simulink model "he6_cres_correlator"  (R2012b) (continued)
== system he6_cres_correlator == (continued)
      mask init:
      | pipeline_init(gcb,'latency',latency);
      settings: AttributesFormatString=Latency = %<latency>
      mask Display:
      | port_label('input', 1, 'd');
      | port_label('output', 1, 'q');
      | disp(['Z^-', num2str(latency)]);
  pipeline22 : Subsystem  AncestorBlock=casper_library_delays/pipeline  mask(pipeline){latency=1}
      mask help:
      | An explicitly laid-out delay line to avoid mapping to SRL16s
      | for use in pipelining to help achieve timing closure.
      mask init:
      | pipeline_init(gcb,'latency',latency);
      settings: AttributesFormatString=Latency = %<latency>
      mask Display:
      | port_label('input', 1, 'd');
      | port_label('output', 1, 'q');
      | disp(['Z^-', num2str(latency)]);
  pipeline23 : Subsystem  AncestorBlock=casper_library_delays/pipeline  mask(pipeline){latency=1}
      mask help:
      | An explicitly laid-out delay line to avoid mapping to SRL16s
      | for use in pipelining to help achieve timing closure.
      mask init:
      | pipeline_init(gcb,'latency',latency);
      settings: AttributesFormatString=Latency = %<latency>
      mask Display:
      | port_label('input', 1, 'd');
      | port_label('output', 1, 'q');
      | disp(['Z^-', num2str(latency)]);
  pipeline24 : Subsystem  AncestorBlock=casper_library_delays/pipeline  mask(pipeline){latency=1}
      mask help:
      | An explicitly laid-out delay line to avoid mapping to SRL16s
      | for use in pipelining to help achieve timing closure.
      mask init:
      | pipeline_init(gcb,'latency',latency);
      settings: AttributesFormatString=Latency = %<latency>
      mask Display:
      | port_label('input', 1, 'd');
      | port_label('output', 1, 'q');
      | disp(['Z^-', num2str(latency)]);
  pipeline25 : Subsystem  AncestorBlock=casper_library_delays/pipeline  mask(pipeline){latency=1}
      mask help:
      | An explicitly laid-out delay line to avoid mapping to SRL16s
      | for use in pipelining to help achieve timing closure.
      mask init:
      | pipeline_init(gcb,'latency',latency);
      settings: AttributesFormatString=Latency = %<latency>
      mask Display:
      | port_label('input', 1, 'd');
      | port_label('output', 1, 'q');
      | disp(['Z^-', num2str(latency)]);
  pipeline26 : Subsystem  AncestorBlock=casper_library_delays/pipeline  mask(pipeline){latency=1}
      mask help:
      | An explicitly laid-out delay line to avoid mapping to SRL16s
      | for use in pipelining to help achieve timing closure.
      mask init:
      | pipeline_init(gcb,'latency',latency);
      settings: AttributesFormatString=Latency = %<latency>
      mask Display:
      | port_label('input', 1, 'd');
      | port_label('output', 1, 'q');
      | disp(['Z^-', num2str(latency)]);
  pipeline27 : Subsystem  AncestorBlock=casper_library_delays/pipeline  mask(pipeline){latency=1}
      mask help:
      | An explicitly laid-out delay line to avoid mapping to SRL16s
      | for use in pipelining to help achieve timing closure.
      mask init:
      | pipeline_init(gcb,'latency',latency);
      settings: AttributesFormatString=Latency = %<latency>
      mask Display:
      | port_label('input', 1, 'd');
      | port_label('output', 1, 'q');
      | disp(['Z^-', num2str(latency)]);
  pipeline28 : Subsystem  AncestorBlock=casper_library_delays/pipeline  mask(pipeline){latency=1}
      mask help:
      | An explicitly laid-out delay line to avoid mapping to SRL16s
      | for use in pipelining to help achieve timing closure.
      mask init:
      | pipeline_init(gcb,'latency',latency);
      settings: AttributesFormatString=Latency = %<latency>
      mask Display:
      | port_label('input', 1, 'd');
      | port_label('output', 1, 'q');
      | disp(['Z^-', num2str(latency)]);
  pipeline29 : Subsystem  AncestorBlock=casper_library_delays/pipeline  mask(pipeline){latency=1}
      mask help:
      | An explicitly laid-out delay line to avoid mapping to SRL16s
      | for use in pipelining to help achieve timing closure.
      mask init:
      | pipeline_init(gcb,'latency',latency);
      settings: AttributesFormatString=Latency = %<latency>
      mask Display:
      | port_label('input', 1, 'd');
      | port_label('output', 1, 'q');
      | disp(['Z^-', num2str(latency)]);
  pipeline3 : Subsystem  AncestorBlock=casper_library_delays/pipeline  mask(pipeline){latency=1}
      mask help:
      | An explicitly laid-out delay line to avoid mapping to SRL16s
      | for use in pipelining to help achieve timing closure.
      mask init:
      | pipeline_init(gcb,'latency',latency);
      settings: AttributesFormatString=Latency = %<latency>
      mask Display:
      | port_label('input', 1, 'd');
      | port_label('output', 1, 'q');
      | disp(['Z^-', num2str(latency)]);
  pipeline30 : Subsystem  AncestorBlock=casper_library_delays/pipeline  mask(pipeline){latency=1}
      mask help:
      | An explicitly laid-out delay line to avoid mapping to SRL16s
      | for use in pipelining to help achieve timing closure.
      mask init:
      | pipeline_init(gcb,'latency',latency);
      settings: AttributesFormatString=Latency = %<latency>
      mask Display:
      | port_label('input', 1, 'd');
      | port_label('output', 1, 'q');
      | disp(['Z^-', num2str(latency)]);
  pipeline31 : Subsystem  AncestorBlock=casper_library_delays/pipeline  mask(pipeline){latency=1}
      mask help:
      | An explicitly laid-out delay line to avoid mapping to SRL16s
      | for use in pipelining to help achieve timing closure.
      mask init:
      | pipeline_init(gcb,'latency',latency);
      settings: AttributesFormatString=Latency = %<latency>
      mask Display:
      | port_label('input', 1, 'd');
      | port_label('output', 1, 'q');
      | disp(['Z^-', num2str(latency)]);
  pipeline32 : Subsystem  AncestorBlock=casper_library_delays/pipeline  mask(pipeline){latency=1}
      mask help:
      | An explicitly laid-out delay line to avoid mapping to SRL16s
      | for use in pipelining to help achieve timing closure.
      mask init:
      | pipeline_init(gcb,'latency',latency);
      settings: AttributesFormatString=Latency = %<latency>
      mask Display:
      | port_label('input', 1, 'd');
      | port_label('output', 1, 'q');
      | disp(['Z^-', num2str(latency)]);
  pipeline33 : Subsystem  AncestorBlock=casper_library_delays/pipeline  mask(pipeline){latency=1}
      mask help:
      | An explicitly laid-out delay line to avoid mapping to SRL16s
      | for use in pipelining to help achieve timing closure.
      mask init:
      | pipeline_init(gcb,'latency',latency);
      settings: AttributesFormatString=Latency = %<latency>
      mask Display:
      | port_label('input', 1, 'd');
      | port_label('output', 1, 'q');
      | disp(['Z^-', num2str(latency)]);
  pipeline34 : Subsystem  AncestorBlock=casper_library_delays/pipeline  mask(pipeline){latency=1}
      mask help:
      | An explicitly laid-out delay line to avoid mapping to SRL16s
      | for use in pipelining to help achieve timing closure.
      mask init:
      | pipeline_init(gcb,'latency',latency);
      settings: AttributesFormatString=Latency = %<latency>
      mask Display:
      | port_label('input', 1, 'd');
      | port_label('output', 1, 'q');
      | disp(['Z^-', num2str(latency)]);
  pipeline35 : Subsystem  AncestorBlock=casper_library_delays/pipeline  mask(pipeline){latency=1}
      mask help:
      | An explicitly laid-out delay line to avoid mapping to SRL16s
      | for use in pipelining to help achieve timing closure.
      mask init:
      | pipeline_init(gcb,'latency',latency);
      settings: AttributesFormatString=Latency = %<latency>
      mask Display:
      | port_label('input', 1, 'd');
      | port_label('output', 1, 'q');
      | disp(['Z^-', num2str(latency)]);
  pipeline36 : Subsystem  AncestorBlock=casper_library_delays/pipeline  mask(pipeline){latency=1}
      mask help:
      | An explicitly laid-out delay line to avoid mapping to SRL16s
      | for use in pipelining to help achieve timing closure.
      mask init:
      | pipeline_init(gcb,'latency',latency);
      settings: AttributesFormatString=Latency = %<latency>
      mask Display:
      | port_label('input', 1, 'd');
      | port_label('output', 1, 'q');
      | disp(['Z^-', num2str(latency)]);
  pipeline37 : Subsystem  AncestorBlock=casper_library_delays/pipeline  mask(pipeline){latency=3}
      mask help:
      | An explicitly laid-out delay line to avoid mapping to SRL16s
      | for use in pipelining to help achieve timing closure.
      mask init:
      | pipeline_init(gcb,'latency',latency);
      settings: AttributesFormatString=Latency = %<latency>
      mask Display:
      | port_label('input', 1, 'd');
      | port_label('output', 1, 'q');
      | disp(['Z^-', num2str(latency)]);
  pipeline4 : Subsystem  AncestorBlock=casper_library_delays/pipeline  mask(pipeline){latency=2}
      mask help:
      | An explicitly laid-out delay line to avoid mapping to SRL16s
      | for use in pipelining to help achieve timing closure.
      mask init:
      | pipeline_init(gcb,'latency',latency);
      settings: AttributesFormatString=Latency = %<latency>
      mask Display:
      | port_label('input', 1, 'd');
      | port_label('output', 1, 'q');
      | disp(['Z^-', num2str(latency)]);
  pipeline6 : Subsystem  AncestorBlock=casper_library_delays/pipeline  mask(pipeline){latency=5}
      mask help:
      | An explicitly laid-out delay line to avoid mapping to SRL16s
      | for use in pipelining to help achieve timing closure.
      mask init:
      | pipeline_init(gcb,'latency',latency);
      settings: AttributesFormatString=Latency = %<latency>
      mask Display:
      | port_label('input', 1, 'd');
      | port_label('output', 1, 'q');
      | disp(['Z^-', num2str(latency)]);
  pipeline7 : Subsystem  AncestorBlock=casper_library_delays/pipeline  mask(pipeline){latency=1}
      mask help:
      | An explicitly laid-out delay line to avoid mapping to SRL16s
      | for use in pipelining to help achieve timing closure.
      mask init:
      | pipeline_init(gcb,'latency',latency);
      settings: AttributesFormatString=Latency = %<latency>
      mask Display:
      | port_label('input', 1, 'd');
      | port_label('output', 1, 'q');
      | disp(['Z^-', num2str(latency)]);
  pipeline8 : Subsystem  AncestorBlock=casper_library_delays/pipeline  mask(pipeline){latency=1}
      mask help:
      | An explicitly laid-out delay line to avoid mapping to SRL16s
      | for use in pipelining to help achieve timing closure.
      mask init:
      | pipeline_init(gcb,'latency',latency);
      settings: AttributesFormatString=Latency = %<latency>
      mask Display:
      | port_label('input', 1, 'd');
      | port_label('output', 1, 'q');
      | disp(['Z^-', num2str(latency)]);
  pipeline9 : Subsystem  AncestorBlock=casper_library_delays/pipeline  mask(pipeline){latency=1}
      mask help:
      | An explicitly laid-out delay line to avoid mapping to SRL16s
      | for use in pipelining to help achieve timing closure.
      mask init:
      | pipeline_init(gcb,'latency',latency);
      settings: AttributesFormatString=Latency = %<latency>
      mask Display:
      | port_label('input', 1, 'd');
      | port_label('output', 1, 'q');
      | disp(['Z^-', num2str(latency)]);
  power0 : casper_library_misc/power  BitWidth=18  add_latency=2  mult_latency=3
      link override power_adder: mode=Addition  use_carryin=off  use_carryout=off  en=off  latency=add_latency  precision=User Defined  arith_type=Unsigned  n_bits=BitWidth * 2  bin_pt=BitWidth * 2 - 1  quantization=Truncate  overflow=Wrap  dbl_ovrd=off  use_behavioral_HDL=on  hw_selection=Fabric  pipelined=off  use_rpm=on
      link override power_adder: xl_area=[0, 0, 0, 0, 0, 0, 0]
  power{1..7} (7 alike) : casper_library_misc/power  BitWidth=18  add_latency=2  mult_latency=3
  rsv0_a : xbsIndex_r4/Constant  const=0  gui_display_data_type=Fixed-point  arith_type=Unsigned  n_bits=64  bin_pt=0  preci_type=Single  exp_width=8  frac_width=24  explicit_period=on  period=1  equ=P=C  opselect=C  inp2=PCIN>>17  opr=+  inp1=P  carry=CIN  dbl_ovrd=off  block_type=constant
  rsv1_a : xbsIndex_r4/Constant  const=0  gui_display_data_type=Fixed-point  arith_type=Unsigned  n_bits=64  bin_pt=0  preci_type=Single  exp_width=8  frac_width=24  explicit_period=on  period=1  equ=P=C  opselect=C  inp2=PCIN>>17  opr=+  inp1=P  carry=CIN  dbl_ovrd=off  block_type=constant
  simple_bram_vacc : Subsystem  AncestorBlock=casper_library_accumulators/simple_bram_vacc  mask(simple_bram_vacc){vec_len=512, arith_type=Unsigned, n_bits=16, bin_pt=0}
      mask prompts: vec_len "Vector length:"; arith_type "Output type:"; n_bits "Bit width (output):"; bin_pt "Binary point (output):"
      mask help: This simple vector accumulator is based on a shift register. It outputs its previous accumulated vector after a new_acc pulse is received.
      mask init:
      | simple_bram_vacc_init(gcb, ...
      |     'vec_len', vec_len, ...
      |     'arith_type', arith_type, ...
      |     'n_bits', n_bits, ...
      |     'bin_pt', bin_pt);
      mask TypeOptions arith_type: Signed | Unsigned
  simple_bram_vacc1 : Subsystem  AncestorBlock=casper_library_accumulators/simple_bram_vacc  mask(simple_bram_vacc){vec_len=512, arith_type=Unsigned, n_bits=16, bin_pt=0}
      mask prompts: vec_len "Vector length:"; arith_type "Output type:"; n_bits "Bit width (output):"; bin_pt "Binary point (output):"
      mask help: This simple vector accumulator is based on a shift register. It outputs its previous accumulated vector after a new_acc pulse is received.
      mask init:
      | simple_bram_vacc_init(gcb, ...
      |     'vec_len', vec_len, ...
      |     'arith_type', arith_type, ...
      |     'n_bits', n_bits, ...
      |     'bin_pt', bin_pt);
      mask TypeOptions arith_type: Signed | Unsigned
  simple_bram_vacc2 : Subsystem  AncestorBlock=casper_library_accumulators/simple_bram_vacc  mask(simple_bram_vacc){vec_len=512, arith_type=Unsigned, n_bits=16, bin_pt=0}
      mask prompts: vec_len "Vector length:"; arith_type "Output type:"; n_bits "Bit width (output):"; bin_pt "Binary point (output):"
      mask help: This simple vector accumulator is based on a shift register. It outputs its previous accumulated vector after a new_acc pulse is received.
      mask init:
      | simple_bram_vacc_init(gcb, ...
      |     'vec_len', vec_len, ...
      |     'arith_type', arith_type, ...
      |     'n_bits', n_bits, ...
      |     'bin_pt', bin_pt);
      mask TypeOptions arith_type: Signed | Unsigned
  simple_bram_vacc3 : Subsystem  AncestorBlock=casper_library_accumulators/simple_bram_vacc  mask(simple_bram_vacc){vec_len=512, arith_type=Unsigned, n_bits=16, bin_pt=0}
      mask prompts: vec_len "Vector length:"; arith_type "Output type:"; n_bits "Bit width (output):"; bin_pt "Binary point (output):"
      mask help: This simple vector accumulator is based on a shift register. It outputs its previous accumulated vector after a new_acc pulse is received.
      mask init:
      | simple_bram_vacc_init(gcb, ...
      |     'vec_len', vec_len, ...
      |     'arith_type', arith_type, ...
      |     'n_bits', n_bits, ...
      |     'bin_pt', bin_pt);
      mask TypeOptions arith_type: Signed | Unsigned
  simple_bram_vacc4 : Subsystem  AncestorBlock=casper_library_accumulators/simple_bram_vacc  mask(simple_bram_vacc){vec_len=512, arith_type=Unsigned, n_bits=16, bin_pt=0}
      mask prompts: vec_len "Vector length:"; arith_type "Output type:"; n_bits "Bit width (output):"; bin_pt "Binary point (output):"
      mask help: This simple vector accumulator is based on a shift register. It outputs its previous accumulated vector after a new_acc pulse is received.
      mask init:
      | simple_bram_vacc_init(gcb, ...
      |     'vec_len', vec_len, ...
      |     'arith_type', arith_type, ...
      |     'n_bits', n_bits, ...
      |     'bin_pt', bin_pt);
      mask TypeOptions arith_type: Signed | Unsigned
  simple_bram_vacc5 : Subsystem  AncestorBlock=casper_library_accumulators/simple_bram_vacc  mask(simple_bram_vacc){vec_len=512, arith_type=Unsigned, n_bits=16, bin_pt=0}
      mask prompts: vec_len "Vector length:"; arith_type "Output type:"; n_bits "Bit width (output):"; bin_pt "Binary point (output):"
      mask help: This simple vector accumulator is based on a shift register. It outputs its previous accumulated vector after a new_acc pulse is received.
      mask init:
      | simple_bram_vacc_init(gcb, ...
      |     'vec_len', vec_len, ...
      |     'arith_type', arith_type, ...
      |     'n_bits', n_bits, ...
      |     'bin_pt', bin_pt);
      mask TypeOptions arith_type: Signed | Unsigned
  simple_bram_vacc6 : Subsystem  AncestorBlock=casper_library_accumulators/simple_bram_vacc  mask(simple_bram_vacc){vec_len=512, arith_type=Unsigned, n_bits=16, bin_pt=0}
      mask prompts: vec_len "Vector length:"; arith_type "Output type:"; n_bits "Bit width (output):"; bin_pt "Binary point (output):"
      mask help: This simple vector accumulator is based on a shift register. It outputs its previous accumulated vector after a new_acc pulse is received.
      mask init:
      | simple_bram_vacc_init(gcb, ...
      |     'vec_len', vec_len, ...
      |     'arith_type', arith_type, ...
      |     'n_bits', n_bits, ...
      |     'bin_pt', bin_pt);
      mask TypeOptions arith_type: Signed | Unsigned
  simple_bram_vacc7 : Subsystem  AncestorBlock=casper_library_accumulators/simple_bram_vacc  mask(simple_bram_vacc){vec_len=512, arith_type=Unsigned, n_bits=16, bin_pt=0}
      mask prompts: vec_len "Vector length:"; arith_type "Output type:"; n_bits "Bit width (output):"; bin_pt "Binary point (output):"
      mask help: This simple vector accumulator is based on a shift register. It outputs its previous accumulated vector after a new_acc pulse is received.
      mask init:
      | simple_bram_vacc_init(gcb, ...
      |     'vec_len', vec_len, ...
      |     'arith_type', arith_type, ...
      |     'n_bits', n_bits, ...
      |     'bin_pt', bin_pt);
      mask TypeOptions arith_type: Signed | Unsigned
  snap_0 : Subsystem
  snap_1 : Subsystem
  sync period -2 : xbsIndex_r4/Constant  const=98302  gui_display_data_type=Fixed-point  arith_type=Unsigned  n_bits=17  bin_pt=0  preci_type=Single  exp_width=8  frac_width=24  explicit_period=off  period=1  equ=P=C  opselect=C  inp2=PCIN>>17  opr=+  inp1=P  carry=CIN  dbl_ovrd=off  block_type=constant
      settings: block_version=10.1.1
  tengbe_a : Subsystem  AncestorBlock=r2daq_blocks/TenGbEWrap  mask(TenGbEWrap){pd_in=3, pd_out=3}
      mask prompts: pd_in "Registers in pipeline delay"; pd_out "Registers out pipeline delay"
      mask help:
      | Wrap 10GbE core and supplies control and status registers internally.
      |
      | Pipeline delay to and from registers are configurable.
      mask init:
      | pd_inst = {...
      |     [gcb '/pd_ctrl'],...
      |     [gcb '/pd_port'],...
      |     [gcb '/pd_ip']};
      | pd_in = get_param(gcb,'pd_in');
      | for ii=1:numel(pd_inst)
      |     set_param(pd_inst{ii},'latency',pd_in);
      | end
      | pd_inst = {...
      |     [gcb '/pd_status']};
      | pd_out = get_param(gcb,'pd_out');
      | for ii=1:numel(pd_inst)
      |     set_param(pd_inst{ii},'latency',pd_out);
      | end
  unix_time0 : Subsystem  Tag=xps:sw_reg  AncestorBlock=xps_library/software_register  mask(swreg){io_dir=From Processor, io_delay=0, sample_period=1, names=reg, bitwidths=32, bin_pts=0, arith_types=0, sim_port=on, show_format=off}
      mask prompts: io_dir "I/O direction"; io_delay "I/O delay"; sample_period "Sample period"; names "Bitfield names [msb...lsb]"; bitwidths "Bitfield widths"; bin_pts "Bitfield binary pts"; arith_types "Bitfield types, ufix=0, fix=1, bool=2"; sim_port "Provide sim input/output?"; show_format "Print format string?"
      mask help: A 32-bit software-accessible register. Can be divided into bitfields of varying widths and types using the fields in the block mask.
      mask init:
      | try
      |     swreg_init(gcb)
      | catch ex
      |     disp(sprintf('%s: %s', gcb, ex.message))
      |     errordlg(ex.message)
      | end
      settings: AttributesFormatString=1input
      mask TypeOptions io_dir: To Processor | From Processor
      mask Callback io_dir:
      | mask_enables = get_param(gcb, 'MaskEnables');
      | mask_names = get_param(gcb, 'MaskNames');
      | if strcmp(get_param(gcb, 'io_dir'), 'To Processor'),
      |     mask_enables{ismember(mask_names, 'sample_period')} = 'off';
      | else
      |     mask_enables{ismember(mask_names, 'sample_period')} = 'on';
      | end
      | set_param(gcb, 'MaskEnables', mask_enables);
  zero : xbsIndex_r4/Constant  const=0  gui_display_data_type=Fixed-point  arith_type=Unsigned  n_bits=20  bin_pt=0  preci_type=Single  exp_width=8  frac_width=24  explicit_period=off  period=1  equ=P=C  opselect=C  inp2=PCIN>>17  opr=+  inp1=P  carry=CIN  dbl_ovrd=off  block_type=constant
  wires:
  pfb_fir_real/1(sync_out) -> pipeline4/1(d)
  pfb_fir_real/2(pol1_out1) -> Shift/1
  pfb_fir_real/3(pol1_out2) -> Shift1/1
  pfb_fir_real/4(pol1_out3) -> Shift2/1
  pfb_fir_real/5(pol1_out4) -> Shift3/1
  Shift/1 -> fft_wideband_real/3(in00)
  Shift1/1 -> fft_wideband_real/4(in01)
  Shift2/1 -> fft_wideband_real/5(in02)
  Shift3/1 -> fft_wideband_real/6(in03)
  pfb_fir_real/16(pol1_out15) -> Shift15/1
  pfb_fir_real/15(pol1_out14) -> Shift14/1
  pfb_fir_real/14(pol1_out13) -> Shift13/1
  pfb_fir_real/13(pol1_out12) -> Shift12/1
  pfb_fir_real/12(pol1_out11) -> Shift10/1
  pfb_fir_real/11(pol1_out10) -> Shift9/1
  pfb_fir_real/10(pol1_out9) -> Shift8/1
  pfb_fir_real/9(pol1_out8) -> Shift11/1
  pfb_fir_real/8(pol1_out7) -> Shift7/1
  pfb_fir_real/7(pol1_out6) -> Shift6/1
  pfb_fir_real/6(pol1_out5) -> Shift5/1
  Shift4/1 -> fft_wideband_real/18(in015)
  Shift15/1 -> fft_wideband_real/17(in014)
  Shift14/1 -> fft_wideband_real/16(in013)
  Shift13/1 -> fft_wideband_real/15(in012)
  Shift12/1 -> fft_wideband_real/14(in011)
  Shift10/1 -> fft_wideband_real/13(in010)
  Shift9/1 -> fft_wideband_real/12(in09)
  Shift8/1 -> fft_wideband_real/11(in08)
  Shift11/1 -> fft_wideband_real/10(in07)
  Shift7/1 -> fft_wideband_real/9(in06)
  Shift6/1 -> fft_wideband_real/8(in05)
  Shift5/1 -> fft_wideband_real/7(in04)
  rsv0_a/1 -> Packetization/4(rsv0)
  rsv1_a/1 -> Packetization/5(rsv1)
  [rst_gt] -> Packetization/2(rst)
  [ut_gt] -> Packetization/3(ut)
  Multipliers/1(Out1) -> pfb_fir_real/2(pol1_in1)
  Multipliers/2(Out2) -> pfb_fir_real/3(pol1_in2)
  Multipliers/3(Out3) -> pfb_fir_real/4(pol1_in3)
  Multipliers/4(Out4) -> pfb_fir_real/5(pol1_in4)
  Multipliers/5(Out5) -> pfb_fir_real/6(pol1_in5)
  Multipliers/6(Out6) -> pfb_fir_real/7(pol1_in6)
  Multipliers/7(Out7) -> pfb_fir_real/8(pol1_in7)
  Multipliers/8(Out8) -> pfb_fir_real/9(pol1_in8)
  Multipliers/9(Out9) -> pfb_fir_real/10(pol1_in9)
  Multipliers/10(Out10) -> pfb_fir_real/11(pol1_in10)
  Multipliers/11(Out11) -> pfb_fir_real/12(pol1_in11)
  Multipliers/12(Out12) -> pfb_fir_real/13(pol1_in12)
  Multipliers/13(Out13) -> pfb_fir_real/14(pol1_in13)
  Multipliers/14(Out14) -> pfb_fir_real/15(pol1_in14)
  Multipliers/15(Out15) -> pfb_fir_real/16(pol1_in15)
  master_ctrl/1(in_reg) -> ctrl_signals/1(master)
  gain_ctrl/1(in_reg) -> ctrl_signals/2(gain)
  fft_ctrl/1(in_reg) -> ctrl_signals/3(fft)
  unix_time0/1(in_reg) -> ctrl_signals/4(ut0)
  ctrl_signals/1(reset) -> [rst_gt]
  ctrl_signals/3(gain_a) -> [gain_a_gt]
  ctrl_signals/7(fft_shift_ab) -> [fft_ab_shift_gt]
  ctrl_signals/4(gain_b) -> Terminator3/1
  ctrl_signals/5(gain_c) -> Terminator1/1
  ctrl_signals/6(gain_d) -> Terminator2/1
  ctrl_signals/8(fft_shift_cd) -> Terminator7/1
  Constant5/1 -> master_ctrl/1(sim_1)
  Constant6/1 -> gain_ctrl/1(sim_1)
  Constant7/1 -> fft_ctrl/1(sim_1)
  Constant8/1 -> unix_time0/1(sim_1)
  ctrl_signals/2(msync) -> [msync_gt]
  ctrl_signals/9(unix_time) -> [ut_gt]
  adc1/17(sync_out) -> Terminator5/1
  ext_sync/1 -> adc0/2(sim_sync)
  if0_data1/1 -> adc1/1(sim_a)
  ext_sync1/1 -> adc1/2(sim_sync)
  master_status/1(sim_out) -> Terminator6/1
  [msync_gt] -> snap_1/1(sync)
  bus_1/1 -> snap_1/2(din)
  [msync_gt] -> snap_0/1(sync)
  bus_0/1 -> snap_0/2(din)
  Concat/1 -> pipeline37/1(d)
  zero/1 -> Concat/1
  cnt_ab/1 -> Concat/2
  [rst_gt] -> cnt_ab/1
  Packetization/4(status) -> Concat/3
  pipeline2/1(q) -> bus_1/1, Multipliers/17(In17)
  pipeline3/1(q) -> bus_1/2, Multipliers/18(In18)
  pipeline7/1(q) -> bus_1/3, Multipliers/19(In19)
  pipeline8/1(q) -> bus_1/4, Multipliers/20(In20)
  pipeline9/1(q) -> bus_1/5, Multipliers/21(In21)
  pipeline10/1(q) -> bus_1/6, Multipliers/22(In22)
  pipeline11/1(q) -> bus_1/7, Multipliers/23(In23)
  pipeline12/1(q) -> bus_1/8, Multipliers/24(In24)
  pipeline13/1(q) -> bus_1/9, Multipliers/25(In25)
  pipeline14/1(q) -> bus_1/10, Multipliers/26(In26)
  pipeline15/1(q) -> bus_1/11, Multipliers/27(In27)
  pipeline16/1(q) -> bus_1/12, Multipliers/28(In28)
  pipeline17/1(q) -> bus_1/13, Multipliers/29(In29)
  pipeline18/1(q) -> bus_1/14, Multipliers/30(In30)
  pipeline19/1(q) -> bus_1/15, Multipliers/31(In31)
  pipeline20/1(q) -> bus_1/16, Multipliers/32(In32)
  pipeline21/1(q) -> bus_0/16, Multipliers/16(In16)
  pipeline22/1(q) -> bus_0/15, Multipliers/15(In15)
  pipeline24/1(q) -> bus_0/13, Multipliers/13(In13)
  pipeline25/1(q) -> bus_0/12, Multipliers/12(In12)
  pipeline26/1(q) -> bus_0/11, Multipliers/11(In11)
  pipeline27/1(q) -> bus_0/10, Multipliers/10(In10)
  pipeline29/1(q) -> bus_0/8, Multipliers/8(In8)
  pipeline30/1(q) -> bus_0/7, Multipliers/7(In7)
  pipeline31/1(q) -> bus_0/6, Multipliers/6(In6)
  pipeline32/1(q) -> bus_0/5, Multipliers/5(In5)
  pipeline33/1(q) -> bus_0/4, Multipliers/4(In4)
  pipeline35/1(q) -> bus_0/2, Multipliers/2(In2)
  pipeline34/1(q) -> bus_0/3, Multipliers/3(In3)
  pipeline37/1(q) -> master_status/1(out_reg)
  fft_wideband_real/10(of) -> Convert8/1
  Convert9/1 -> cnt_ab/2
  Convert8/1 -> Convert9/1
  Packetization/1(tx_eof1) -> tengbe_a/1(tx_eof)
  Packetization/2(tx_valid1) -> tengbe_a/2(tx_valid)
  MSB/1 -> edge_detect_1/1
  simple_bram_vacc/1(dout) -> bus/1(in1)
  simple_bram_vacc1/1(dout) -> bus/2(in2)
  simple_bram_vacc2/1(dout) -> bus/3(in3)
  simple_bram_vacc3/1(dout) -> bus/4(in4)
  simple_bram_vacc4/1(dout) -> bus/5(in5)
  simple_bram_vacc5/1(dout) -> bus/6(in6)
  simple_bram_vacc6/1(dout) -> bus/7(in7)
  simple_bram_vacc/2(valid) -> edge_detect_3/1
  bus/1(bus_out) -> pipeline6/1(d)
  simple_bram_vacc1/2(valid) -> Terminator9/1
  simple_bram_vacc2/2(valid) -> Terminator10/1
  simple_bram_vacc3/2(valid) -> Terminator11/1
  simple_bram_vacc4/2(valid) -> Terminator12/1
  simple_bram_vacc5/2(valid) -> Terminator13/1
  simple_bram_vacc6/2(valid) -> Terminator14/1
  simple_bram_vacc7/2(valid) -> Terminator15/1
  pfb_fir_real/17(pol1_out16) -> Shift4/1
  Multipliers/16(Out16) -> pfb_fir_real/17(pol1_in16)
  pipeline4/1(q) -> fft_wideband_real/1(sync)
  power0/1 -> Slice1/1
  power1/1 -> Slice2/1
  power2/1 -> Slice3/1
  power3/1 -> Slice4/1
  power4/1 -> Slice5/1
  power5/1 -> Slice6/1
  power6/1 -> Slice7/1
  power7/1 -> Slice8/1
  simple_bram_vacc7/1(dout) -> bus/8(in8)
  adc0/1(a0) -> Renormalize/1(In1)
  Renormalize/1(Out1) -> pipeline36/1(d)
  adc0/2(a1) -> Renormalize1/1(In1)
  Renormalize1/1(Out1) -> pipeline35/1(d)
  adc0/3(a2) -> Renormalize2/1(In1)
  Renormalize2/1(Out1) -> pipeline34/1(d)
  Renormalize3/1(Out1) -> pipeline33/1(d)
  adc0/4(a3) -> Renormalize3/1(In1)
  Renormalize4/1(Out1) -> pipeline32/1(d)
  adc0/5(a4) -> Renormalize4/1(In1)
  adc0/6(a5) -> Renormalize5/1(In1)
  Renormalize5/1(Out1) -> pipeline31/1(d)
  Renormalize6/1(Out1) -> pipeline30/1(d)
  adc0/7(a6) -> Renormalize6/1(In1)
  adc0/8(a7) -> Renormalize7/1(In1)
  Renormalize7/1(Out1) -> pipeline29/1(d)
  adc0/9(a8) -> Renormalize8/1(In1)
  Renormalize8/1(Out1) -> pipeline28/1(d)
  Renormalize9/1(Out1) -> pipeline27/1(d)
  adc0/10(a9) -> Renormalize9/1(In1)
  Renormalize10/1(Out1) -> pipeline26/1(d)
  adc0/11(a10) -> Renormalize10/1(In1)
  adc0/12(a11) -> Renormalize11/1(In1)
  Renormalize11/1(Out1) -> pipeline25/1(d)
  Renormalize12/1(Out1) -> pipeline24/1(d)
  adc0/13(a12) -> Renormalize12/1(In1)
  adc0/14(a13) -> Renormalize13/1(In1)
  Renormalize13/1(Out1) -> pipeline23/1(d)
  Renormalize14/1(Out1) -> pipeline22/1(d)
  adc0/15(a14) -> Renormalize14/1(In1)
  adc0/16(a15) -> Renormalize15/1(In1)
  Renormalize15/1(Out1) -> pipeline21/1(d)
  adc1/1(a0) -> Renormalize16/1(In1)
  Renormalize16/1(Out1) -> pipeline2/1(d)
  Renormalize17/1(Out1) -> pipeline3/1(d)
  adc1/2(a1) -> Renormalize17/1(In1)
  Renormalize18/1(Out1) -> pipeline7/1(d)
  adc1/3(a2) -> Renormalize18/1(In1)
  adc1/4(a3) -> Renormalize19/1(In1)
  Renormalize19/1(Out1) -> pipeline8/1(d)
  Renormalize20/1(Out1) -> pipeline9/1(d)
  adc1/5(a4) -> Renormalize20/1(In1)
  adc1/6(a5) -> Renormalize21/1(In1)
  Renormalize21/1(Out1) -> pipeline10/1(d)
  Renormalize22/1(Out1) -> pipeline11/1(d)
  adc1/7(a6) -> Renormalize22/1(In1)
  adc1/8(a7) -> Renormalize23/1(In1)
  Renormalize23/1(Out1) -> pipeline12/1(d)
  Renormalize24/1(Out1) -> pipeline13/1(d)
  adc1/9(a8) -> Renormalize24/1(In1)
  adc1/10(a9) -> Renormalize25/1(In1)
  Renormalize25/1(Out1) -> pipeline14/1(d)
  Renormalize26/1(Out1) -> pipeline15/1(d)
  adc1/11(a10) -> Renormalize26/1(In1)
  adc1/12(a11) -> Renormalize27/1(In1)
  Renormalize27/1(Out1) -> pipeline16/1(d)
  Renormalize28/1(Out1) -> pipeline17/1(d)
  adc1/13(a12) -> Renormalize28/1(In1)
  adc1/14(a13) -> Renormalize29/1(In1)
  Renormalize29/1(Out1) -> pipeline18/1(d)
  Renormalize30/1(Out1) -> pipeline19/1(d)
  Renormalize31/1(Out1) -> pipeline20/1(d)
  adc1/15(a14) -> Renormalize30/1(In1)
  adc1/16(a15) -> Renormalize31/1(In1)
  Delay1/1 -> Delay/1
  Delay3/1 -> Delay2/1
  Delay2/1 -> power1/1
  Delay4/1 -> power2/1
  Delay5/1 -> Delay4/1
  Delay7/1 -> Delay6/1
  Delay6/1 -> power3/1
  Delay8/1 -> power4/1
  Delay9/1 -> Delay8/1
  Delay11/1 -> Delay10/1
  Delay10/1 -> power5/1
  Delay12/1 -> power6/1
  Delay13/1 -> Delay12/1
  Delay14/1 -> power7/1
  Delay15/1 -> Delay14/1
  Slice1/1 -> Shift16/1
  Slice2/1 -> Shift17/1
  Slice3/1 -> Shift18/1
  Slice4/1 -> Shift19/1
  Slice5/1 -> Shift20/1
  Slice6/1 -> Shift21/1
  Slice7/1 -> Shift22/1
  Slice8/1 -> Shift23/1
  if0_data/1 -> adc0/1(sim_a)
  pipeline36/1(q) -> bus_0/1, Multipliers/1(In1)
  pipeline28/1(q) -> bus_0/9, Multipliers/9(In9)
  pipeline23/1(q) -> bus_0/14, Multipliers/14(In14)
  Packetization/3(tx_data1) -> tengbe_a/3(tx_data)
  Logical2/1 -> 12-bit free running/1
  [rst_gt] -> Logical2/1
  pipeline1/1(q) -> Logical2/2
  fft_wideband_real/1(sync_out) -> pipeline1/1(d)
  edge_detect_1/1 -> simple_bram_vacc2/1(new_acc), simple_bram_vacc3/1(new_acc), simple_bram_vacc4/1(new_acc), simple_bram_vacc5/1(new_acc), simple_bram_vacc6/1(new_acc), simple_bram_vacc7/1(new_acc), simple_bram_vacc1/1(new_acc), simple_bram_vacc/1(new_acc)
  pipeline6/1(q) -> Packetization/6(fdata)
  [rst_gt] -> Logical1/2
  edge_detect_2/1 -> pfb_fir_real/1(sync)
  Logical1/1 -> 17-bit free-running count/1
  Relational/1 -> Logical3/1, Logical1/1
  17-bit free-running count/1 -> Relational/2
  sync period -2/1 -> Relational/1
  Logical3/1 -> edge_detect_2/1
  adc0/17(sync_out) -> Logical3/2
  edge_detect_3/1 -> Packetization/1(fsync_in)
  Delay/1 -> power0/1
  12-bit free running/1 -> MSB/1
  fft_wideband_real/2(out00) -> Delay1/1
  fft_wideband_real/3(out01) -> Delay3/1
  fft_wideband_real/4(out02) -> Delay5/1
  fft_wideband_real/5(out03) -> Delay7/1
  fft_wideband_real/6(out04) -> Delay9/1
  fft_wideband_real/7(out05) -> Delay11/1
  fft_wideband_real/8(out06) -> Delay13/1
  fft_wideband_real/9(out07) -> Delay15/1
  Shift16/1 -> simple_bram_vacc/2(din)
  Shift17/1 -> simple_bram_vacc1/2(din)
  Shift18/1 -> simple_bram_vacc2/2(din)
  Shift19/1 -> simple_bram_vacc3/2(din)
  Shift20/1 -> simple_bram_vacc4/2(din)
  Shift21/1 -> simple_bram_vacc5/2(din)
  Shift22/1 -> simple_bram_vacc6/2(din)
  Shift23/1 -> simple_bram_vacc7/2(din)
  [fft_ab_shift_gt] -> fft_wideband_real/2(shift)
